simulink model "MCVT_Integration"  (R2020a)
settings: Solver=ode8  StartTime=0.0  StopTime=T_end  FixedStep=dt  RelTol=1e-3  EnableMultiTasking=on
values from neighbouring files (symbols this model uses):
  assumed_power_voltage = 1.25e2    (= 125)    [MCVT_Input_File.m]    (also in MCVT_Run_QuickCheck.m)
  battery_power_toggle = 1    [MCVT_Input_File.m]    (also in MCVT_Run_QuickCheck.m)
  battery_trickle_charge_param = 0.1    [MCVT_Input_File.m]    (also in MCVT_Run_QuickCheck.m)
  battery_trickle_leakage_param = 0.01    [MCVT_Input_File.m]    (also in MCVT_Run_QuickCheck.m)
  damage_case_id = 3    [MCVT_Input_File.m]    (also in MCVT_Run_QuickCheck.m = 1)
  dt = 1/fs    (= 0.001)    [MCVT_Run_QuickCheck.m]    (also in MCVT_Sim_Set.m)
  dt_2 = 1/fs_2    (= 0.001)    [MCVT_Run_QuickCheck.m]    (also in MCVT_Sim_Set.m)
  dt_3 = 1/fs_3    (= 0.001)    [MCVT_Run_QuickCheck.m]    (also in MCVT_Sim_Set.m)
  dt_5 = 1/fs_5    (= 0.005)    [MCVT_Run_QuickCheck.m]    (also in MCVT_Sim_Set.m)
  dt_8 = 1/fs_8    (= 0.05)    [MCVT_Run_QuickCheck.m]    (also in MCVT_Sim_Set.m)
  dt_9 = 1/fs_9    (= 0.001)    [MCVT_Run_QuickCheck.m]    (also in MCVT_Sim_Set.m)
  dust_met_duration = 3    [MCVT_Input_File.m]    (also in MCVT_Run_QuickCheck.m = 2)
  ECLSS_indoor_set_temperature = 298.15    [MCVT_Input_File.m]    (also in MCVT_Run_QuickCheck.m)
  energy_store_max_o = 150    [MCVT_Input_File.m]    (also in MCVT_Run_QuickCheck.m)
  energy_store_o = 120    [MCVT_Input_File.m]    (also in MCVT_Run_QuickCheck.m)
  fs = 1000    [MCVT_Run_QuickCheck.m]    (also in MCVT_Sim_Set.m)
  gain_charge_param = 1    [MCVT_Input_File.m]    (also in MCVT_Run_QuickCheck.m)
  ground_temperature = 373    [MCVT_Habitat_Design_Input_File.m]    (also in MCVT_Run_QuickCheck.m)
  I_max_charge = 800    [MCVT_Input_File.m]    (also in MCVT_Run_QuickCheck.m)
  I_max_discharge = 800    [MCVT_Input_File.m]    (also in MCVT_Run_QuickCheck.m)
  initial_pressure = 1e5    (= 100000)    [MCVT_Input_File.m]    (also in MCVT_Run_QuickCheck.m)
  initial_temperature = 295    [MCVT_Input_File.m]    (also in MCVT_Run_QuickCheck.m)
  interior_leak_rate = 0.00415    [MCVT_Input_File.m]    (also in MCVT_Run_QuickCheck.m)
  launch_dust_accum_start_time = 10    [MCVT_Input_File.m]    (also in MCVT_Run_QuickCheck.m = 2)
  launch_dust_accum_stop_time = 12    [MCVT_Input_File.m]    (also in MCVT_Run_QuickCheck.m = 3)
  meteorite_impact_time = 2    [MCVT_Input_File.m]    (also in MCVT_Run_QuickCheck.m = 1)
  nuclear_power_toggle = 1    [MCVT_Input_File.m]    (also in MCVT_Run_QuickCheck.m)
  print_interval = 30    [MCVT_Input_File.m]
  SOC_lower_thres = 0.5    [MCVT_Input_File.m]    (also in MCVT_Run_QuickCheck.m)
  SOC_upper_thres = 0.9    [MCVT_Input_File.m]    (also in MCVT_Run_QuickCheck.m)
  solar_power_toggle = 1    [MCVT_Input_File.m]    (also in MCVT_Run_QuickCheck.m)
  space_temperature = 4    [MCVT_Habitat_Design_Input_File.m]    (also in MCVT_Run_QuickCheck.m)
  Start_angle = 90    [MCVT_Input_File.m]    (also in MCVT_Run_QuickCheck.m)
undefined symbols (not defined in the file or in its folder): A_flow_restrictor, ACTIVITY_LOOKUP_TABLE, air, Aref, Bmap, Bmap2, Bmap3, comms, DiscIntNeverNeededParam, DmgSPL, dust_parameters, ECLSS_dust_damage_repair_time, ECLSS_heater_capacity, ECLSS_IPS_restrictor, ECLSS_IPS_setpointPressure, ECLSS_paint_damage_repair_time, ECLSS_radiatorLoop_discreteNumber, ECLSS_Vst, emptyActivity, expected_heat_add, FI, habitat, HIEM2SMM, HIEM2STM, HIEMDamageElements, impact_numbering, initial_SPLT_damage_index, input_air, input_Cond, input_CP, input_discretization, input_EV, input_Evap, input_radiatorLoop, K_ECLSS_dust_single, K_ECLSS_paint_single, K_Structure_damage_single, Kref, kW_to_W, meteoroidDustDep, mFRF, ndelays, nominalDustDep, noverlap, nuclear_toggle_gain, output_limits, panel_paint0, Ps0, reference_temperature, RepairTime_ECLSS_factor, Sensor_Location_SMM, SMM_T0, SMM_T1, SMM_T2, SMM_T3, SMM_T4, SMM_T4_0, SMM_T4_1, SMM_T4_2, solar_FDD_K ... (+73)
libraries: neural x350, simulink x39, xpcutilitieslib x7, xpcrtudplib x7, dspstat3 x1
(part 37 of 43)

== system MCVT_Integration/Sys3 - Power System/Power Generation Subsystem1/Nuclear Power Generation/SP-100 Power Generation/	Power Generation/Nominal Power/Sensor Models/Cold Junction Temperature Sensing Model/ Resistance Temperature Detector/Switch ON//OFF Control Block ==
  In1 : Inport
  In2 : Inport  Port=2
  Multiport Switch1 : MultiPortSwitch  Inputs=2
  Switch OFF : Subsystem
  Switch ON : Subsystem
  Out1 : Outport  VectorParamsAs1DForOutWhenUnconnected=off
  wires:
  Multiport Switch1/1 -> Out1/1
  In2/1 -> Switch ON/1(In1), Switch OFF/1(In1)
  In1/1 -> Multiport Switch1/1
  Switch ON/1(ON) -> Multiport Switch1/2
  Switch OFF/1(OFF) -> Multiport Switch1/3

== system MCVT_Integration/Sys3 - Power System/Power Generation Subsystem1/Nuclear Power Generation/SP-100 Power Generation/	Power Generation/Nominal Power/Sensor Models/Cold Junction Temperature Sensing Model1/ Resistance Temperature Detector/RTD with Self-heat Error ==
  Resistance Change with Error : Inport
  " Current in Circuit" : Constant  Value=0.001
  MATLAB Function : MATLAB Function  TreatAsAtomicUnit=on
  Voltage Output with Error : Outport  VectorParamsAs1DForOutWhenUnconnected=off
  wires:
  Resistance Change with Error/1 -> MATLAB Function/2(R)
  MATLAB Function/1(U) -> Voltage Output with Error/1
  " Current in Circuit"/1 -> MATLAB Function/1(I)

== system MCVT_Integration/Sys3 - Power System/Power Generation Subsystem1/Nuclear Power Generation/SP-100 Power Generation/	Power Generation/Nominal Power/Sensor Models/Cold Junction Temperature Sensing Model1/ Resistance Temperature Detector/RTD without Self-heat Error ==
  Resistance Change without Error : Inport
  " Current in Circuit" : Constant  Value=0.001
  MATLAB Function : MATLAB Function  TreatAsAtomicUnit=on
  Current in Circuit : Outport  VectorParamsAs1DForOutWhenUnconnected=off
  Voltage Output without Error : Outport  Port=2  VectorParamsAs1DForOutWhenUnconnected=off
  wires:
  Resistance Change without Error/1 -> MATLAB Function/2(R)
  MATLAB Function/2(U) -> Voltage Output without Error/1
  MATLAB Function/1(I) -> Current in Circuit/1
  " Current in Circuit"/1 -> MATLAB Function/1("I ")

== system MCVT_Integration/Sys3 - Power System/Power Generation Subsystem1/Nuclear Power Generation/SP-100 Power Generation/	Power Generation/Nominal Power/Sensor Models/Cold Junction Temperature Sensing Model1/ Resistance Temperature Detector/Self-heat Calculation ==
  note: Power = I 2 *R
  Real Temperature : Inport
  Resistance Change without Error : Inport  Port=2
  Current in Circuit : Inport  Port=3
  "  T vs R " : MATLAB Function  TreatAsAtomicUnit=on
  Add1 : Sum
  Divide1 : Product  Inputs=*/
  Gain : Gain  Gain=1000
  Product : Product
  Self Heating Constant (mW/℃) : Constant  Value=15
  Square : Math  Operator=square  SignedPower=on  SaturateOnIntegerOverflow=on
  Resistance Change with Error : Outport  VectorParamsAs1DForOutWhenUnconnected=off
  Self-heat Error : Outport  Port=2  VectorParamsAs1DForOutWhenUnconnected=off
  wires:
  Self Heating Constant (mW/℃)/1 -> Divide1/2
  Product/1 -> Divide1/1
  Add1/1 -> "  T vs R "/1(T)
  Divide1/1 -> Self-heat Error/1, Add1/2  "self_heat_error"
  Resistance Change without Error/1 -> Product/1
  "  T vs R "/1(R) -> Resistance Change with Error/1
  Real Temperature/1 -> Add1/1
  Gain/1 -> Square/1
  Square/1 -> Product/2
  Current in Circuit/1 -> Gain/1

== system MCVT_Integration/Sys3 - Power System/Power Generation Subsystem1/Nuclear Power Generation/SP-100 Power Generation/	Power Generation/Nominal Power/Sensor Models/Cold Junction Temperature Sensing Model1/ Resistance Temperature Detector/Switch ON//OFF Control Block ==
  In1 : Inport
  In2 : Inport  Port=2
  Multiport Switch1 : MultiPortSwitch  Inputs=2
  Switch OFF : Subsystem
  Switch ON : Subsystem
  Out1 : Outport  VectorParamsAs1DForOutWhenUnconnected=off
  wires:
  Multiport Switch1/1 -> Out1/1
  In2/1 -> Switch ON/1(In1), Switch OFF/1(In1)
  In1/1 -> Multiport Switch1/1
  Switch ON/1(ON) -> Multiport Switch1/2
  Switch OFF/1(OFF) -> Multiport Switch1/3

== system MCVT_Integration/Sys3 - Power System/Power Generation Subsystem1/Nuclear Power Generation/SP-100 Power Generation/	Power Generation/Nominal Power/Sensor Models/Cold Junction Temperature Sensing Model2/ Resistance Temperature Detector/RTD with Self-heat Error ==
  Resistance Change with Error : Inport
  " Current in Circuit" : Constant  Value=0.001
  MATLAB Function : MATLAB Function  TreatAsAtomicUnit=on
  Voltage Output with Error : Outport  VectorParamsAs1DForOutWhenUnconnected=off
  wires:
  " Current in Circuit"/1 -> MATLAB Function/1(I)
  MATLAB Function/1(U) -> Voltage Output with Error/1
  Resistance Change with Error/1 -> MATLAB Function/2(R)

== system MCVT_Integration/Sys3 - Power System/Power Generation Subsystem1/Nuclear Power Generation/SP-100 Power Generation/	Power Generation/Nominal Power/Sensor Models/Cold Junction Temperature Sensing Model2/ Resistance Temperature Detector/RTD without Self-heat Error ==
  Resistance Change without Error : Inport
  " Current in Circuit" : Constant  Value=0.001
  MATLAB Function : MATLAB Function  TreatAsAtomicUnit=on
  Current in Circuit : Outport  VectorParamsAs1DForOutWhenUnconnected=off
  Voltage Output without Error : Outport  Port=2  VectorParamsAs1DForOutWhenUnconnected=off
  wires:
  " Current in Circuit"/1 -> MATLAB Function/1("I ")
  MATLAB Function/1(I) -> Current in Circuit/1
  MATLAB Function/2(U) -> Voltage Output without Error/1
  Resistance Change without Error/1 -> MATLAB Function/2(R)

== system MCVT_Integration/Sys3 - Power System/Power Generation Subsystem1/Nuclear Power Generation/SP-100 Power Generation/	Power Generation/Nominal Power/Sensor Models/Cold Junction Temperature Sensing Model2/ Resistance Temperature Detector/Self-heat Calculation ==
  note: Power = I 2 *R
  Real Temperature : Inport
  Resistance Change without Error : Inport  Port=2
  Current in Circuit : Inport  Port=3
  "  T vs R " : MATLAB Function  TreatAsAtomicUnit=on
  Add1 : Sum
  Divide1 : Product  Inputs=*/
  Gain : Gain  Gain=1000
  Product : Product
  Self Heating Constant (mW/℃) : Constant  Value=15
  Square : Math  Operator=square  SignedPower=on  SaturateOnIntegerOverflow=on
  Resistance Change with Error : Outport  VectorParamsAs1DForOutWhenUnconnected=off
  Self-heat Error : Outport  Port=2  VectorParamsAs1DForOutWhenUnconnected=off
  wires:
  Current in Circuit/1 -> Gain/1
  Square/1 -> Product/2
  Gain/1 -> Square/1
  Real Temperature/1 -> Add1/1
  "  T vs R "/1(R) -> Resistance Change with Error/1
  Resistance Change without Error/1 -> Product/1
  Divide1/1 -> Add1/2, Self-heat Error/1
  Add1/1 -> "  T vs R "/1(T)
  Product/1 -> Divide1/1
  Self Heating Constant (mW/℃)/1 -> Divide1/2

== system MCVT_Integration/Sys3 - Power System/Power Generation Subsystem1/Nuclear Power Generation/SP-100 Power Generation/	Power Generation/Nominal Power/Sensor Models/Cold Junction Temperature Sensing Model2/ Resistance Temperature Detector/Switch ON//OFF Control Block ==
  In1 : Inport
  In2 : Inport  Port=2
  Multiport Switch1 : MultiPortSwitch  Inputs=2
  Switch OFF : Subsystem
  Switch ON : Subsystem
  Out1 : Outport  VectorParamsAs1DForOutWhenUnconnected=off
  wires:
  Switch OFF/1(OFF) -> Multiport Switch1/3
  Switch ON/1(ON) -> Multiport Switch1/2
  In1/1 -> Multiport Switch1/1
  In2/1 -> Switch OFF/1(In1), Switch ON/1(In1)
  Multiport Switch1/1 -> Out1/1

== system MCVT_Integration/Sys3 - Power System/Power Generation Subsystem1/Nuclear Power Generation/SP-100 Power Generation/	Power Generation/Nominal Power/Sensor Models/Cold Junction Temperature Sensing Model3/ Resistance Temperature Detector/RTD with Self-heat Error ==
  Resistance Change with Error : Inport
  " Current in Circuit" : Constant  Value=0.001
  MATLAB Function : MATLAB Function  TreatAsAtomicUnit=on
  Voltage Output with Error : Outport  VectorParamsAs1DForOutWhenUnconnected=off
  wires:
  Resistance Change with Error/1 -> MATLAB Function/2(R)
  MATLAB Function/1(U) -> Voltage Output with Error/1
  " Current in Circuit"/1 -> MATLAB Function/1(I)

== system MCVT_Integration/Sys3 - Power System/Power Generation Subsystem1/Nuclear Power Generation/SP-100 Power Generation/	Power Generation/Nominal Power/Sensor Models/Cold Junction Temperature Sensing Model3/ Resistance Temperature Detector/RTD without Self-heat Error ==
  Resistance Change without Error : Inport
  " Current in Circuit" : Constant  Value=0.001
  MATLAB Function : MATLAB Function  TreatAsAtomicUnit=on
  Current in Circuit : Outport  VectorParamsAs1DForOutWhenUnconnected=off
  Voltage Output without Error : Outport  Port=2  VectorParamsAs1DForOutWhenUnconnected=off
  wires:
  Resistance Change without Error/1 -> MATLAB Function/2(R)
  MATLAB Function/2(U) -> Voltage Output without Error/1
  MATLAB Function/1(I) -> Current in Circuit/1
  " Current in Circuit"/1 -> MATLAB Function/1("I ")

== system MCVT_Integration/Sys3 - Power System/Power Generation Subsystem1/Nuclear Power Generation/SP-100 Power Generation/	Power Generation/Nominal Power/Sensor Models/Cold Junction Temperature Sensing Model3/ Resistance Temperature Detector/Self-heat Calculation ==
  note: Power = I 2 *R
  Real Temperature : Inport
  Resistance Change without Error : Inport  Port=2
  Current in Circuit : Inport  Port=3
  "  T vs R " : MATLAB Function  TreatAsAtomicUnit=on
  Add1 : Sum
  Divide1 : Product  Inputs=*/
  Gain : Gain  Gain=1000
  Product : Product
  Self Heating Constant (mW/℃) : Constant  Value=15
  Square : Math  Operator=square  SignedPower=on  SaturateOnIntegerOverflow=on
  Resistance Change with Error : Outport  VectorParamsAs1DForOutWhenUnconnected=off
  Self-heat Error : Outport  Port=2  VectorParamsAs1DForOutWhenUnconnected=off
  wires:
  Self Heating Constant (mW/℃)/1 -> Divide1/2
  Product/1 -> Divide1/1
  Add1/1 -> "  T vs R "/1(T)
  Divide1/1 -> Self-heat Error/1, Add1/2
  Resistance Change without Error/1 -> Product/1
  "  T vs R "/1(R) -> Resistance Change with Error/1
  Real Temperature/1 -> Add1/1
  Gain/1 -> Square/1
  Square/1 -> Product/2
  Current in Circuit/1 -> Gain/1

== system MCVT_Integration/Sys3 - Power System/Power Generation Subsystem1/Nuclear Power Generation/SP-100 Power Generation/	Power Generation/Nominal Power/Sensor Models/Cold Junction Temperature Sensing Model3/ Resistance Temperature Detector/Switch ON//OFF Control Block ==
  In1 : Inport
  In2 : Inport  Port=2
  Multiport Switch1 : MultiPortSwitch  Inputs=2
  Switch OFF : Subsystem
  Switch ON : Subsystem
  Out1 : Outport  VectorParamsAs1DForOutWhenUnconnected=off
  wires:
  Multiport Switch1/1 -> Out1/1
  In2/1 -> Switch ON/1(In1), Switch OFF/1(In1)
  In1/1 -> Multiport Switch1/1
  Switch ON/1(ON) -> Multiport Switch1/2
  Switch OFF/1(OFF) -> Multiport Switch1/3

== system MCVT_Integration/Sys3 - Power System/Power Generation Subsystem1/Nuclear Power Generation/SP-100 Power Generation/	Power Generation/Nominal Power/Sensor Models/Cold Junction Temperature Sensing Model4/ Resistance Temperature Detector/RTD with Self-heat Error ==
  Resistance Change with Error : Inport
  " Current in Circuit" : Constant  Value=0.001
  MATLAB Function : MATLAB Function  TreatAsAtomicUnit=on
  Voltage Output with Error : Outport  VectorParamsAs1DForOutWhenUnconnected=off
  wires:
  " Current in Circuit"/1 -> MATLAB Function/1(I)
  MATLAB Function/1(U) -> Voltage Output with Error/1
  Resistance Change with Error/1 -> MATLAB Function/2(R)

== system MCVT_Integration/Sys3 - Power System/Power Generation Subsystem1/Nuclear Power Generation/SP-100 Power Generation/	Power Generation/Nominal Power/Sensor Models/Cold Junction Temperature Sensing Model4/ Resistance Temperature Detector/RTD without Self-heat Error ==
  Resistance Change without Error : Inport
  " Current in Circuit" : Constant  Value=0.001
  MATLAB Function : MATLAB Function  TreatAsAtomicUnit=on
  Current in Circuit : Outport  VectorParamsAs1DForOutWhenUnconnected=off
  Voltage Output without Error : Outport  Port=2  VectorParamsAs1DForOutWhenUnconnected=off
  wires:
  " Current in Circuit"/1 -> MATLAB Function/1("I ")
  MATLAB Function/1(I) -> Current in Circuit/1
  MATLAB Function/2(U) -> Voltage Output without Error/1
  Resistance Change without Error/1 -> MATLAB Function/2(R)

== system MCVT_Integration/Sys3 - Power System/Power Generation Subsystem1/Nuclear Power Generation/SP-100 Power Generation/	Power Generation/Nominal Power/Sensor Models/Cold Junction Temperature Sensing Model4/ Resistance Temperature Detector/Self-heat Calculation ==
  note: Power = I 2 *R
  Real Temperature : Inport
  Resistance Change without Error : Inport  Port=2
  Current in Circuit : Inport  Port=3
  "  T vs R " : MATLAB Function  TreatAsAtomicUnit=on
  Add1 : Sum
  Divide1 : Product  Inputs=*/
  Gain : Gain  Gain=1000
  Product : Product
  Self Heating Constant (mW/℃) : Constant  Value=15
  Square : Math  Operator=square  SignedPower=on  SaturateOnIntegerOverflow=on
  Resistance Change with Error : Outport  VectorParamsAs1DForOutWhenUnconnected=off
  Self-heat Error : Outport  Port=2  VectorParamsAs1DForOutWhenUnconnected=off
  wires:
  Current in Circuit/1 -> Gain/1
  Square/1 -> Product/2
  Gain/1 -> Square/1
  Real Temperature/1 -> Add1/1
  "  T vs R "/1(R) -> Resistance Change with Error/1
  Resistance Change without Error/1 -> Product/1
  Divide1/1 -> Add1/2, Self-heat Error/1
  Add1/1 -> "  T vs R "/1(T)
  Product/1 -> Divide1/1
  Self Heating Constant (mW/℃)/1 -> Divide1/2

== system MCVT_Integration/Sys3 - Power System/Power Generation Subsystem1/Nuclear Power Generation/SP-100 Power Generation/	Power Generation/Nominal Power/Sensor Models/Cold Junction Temperature Sensing Model4/ Resistance Temperature Detector/Switch ON//OFF Control Block ==
  In1 : Inport
  In2 : Inport  Port=2
  Multiport Switch1 : MultiPortSwitch  Inputs=2
  Switch OFF : Subsystem
  Switch ON : Subsystem
  Out1 : Outport  VectorParamsAs1DForOutWhenUnconnected=off
  wires:
  Switch OFF/1(OFF) -> Multiport Switch1/3
  Switch ON/1(ON) -> Multiport Switch1/2
  In1/1 -> Multiport Switch1/1
  In2/1 -> Switch OFF/1(In1), Switch ON/1(In1)
  Multiport Switch1/1 -> Out1/1

== system MCVT_Integration/Sys3 - Power System/Power Generation Subsystem1/Nuclear Power Generation/SP-100 Power Generation/	Power Generation/Sensor Models/Cold Junction Temperature Sensing Model/ Resistance Temperature Detector/Switch ON//OFF Control Block/Switch OFF ==
  In1 : Inport
  Clock1 : Clock
  MATLAB Function1 : MATLAB Function  TreatAsAtomicUnit=on
  No Signal : Scope
  OFF : Outport  VectorParamsAs1DForOutWhenUnconnected=off
  wires:
  In1/1 -> MATLAB Function1/1(u)
  Clock1/1 -> MATLAB Function1/2(Time)
  MATLAB Function1/1(y) -> OFF/1, No Signal/1

== system MCVT_Integration/Sys3 - Power System/Power Generation Subsystem1/Nuclear Power Generation/SP-100 Power Generation/	Power Generation/Sensor Models/Cold Junction Temperature Sensing Model/ Resistance Temperature Detector/Switch ON//OFF Control Block/Switch ON ==
  In1 : Inport
  Gain2 : Gain
  Signal Received : Scope
  ON : Outport  VectorParamsAs1DForOutWhenUnconnected=off
  wires:
  Gain2/1 -> ON/1, Signal Received/1
  In1/1 -> Gain2/1

== system MCVT_Integration/Sys3 - Power System/Power Generation Subsystem1/Nuclear Power Generation/SP-100 Power Generation/	Power Generation/Sensor Models/Cold Junction Temperature Sensing Model1/ Resistance Temperature Detector/Switch ON//OFF Control Block/Switch OFF ==
  In1 : Inport
  Clock1 : Clock
  MATLAB Function1 : MATLAB Function  TreatAsAtomicUnit=on
  No Signal : Scope
  OFF : Outport  VectorParamsAs1DForOutWhenUnconnected=off
  wires:
  In1/1 -> MATLAB Function1/1(u)
  Clock1/1 -> MATLAB Function1/2(Time)
  MATLAB Function1/1(y) -> OFF/1, No Signal/1

== system MCVT_Integration/Sys3 - Power System/Power Generation Subsystem1/Nuclear Power Generation/SP-100 Power Generation/	Power Generation/Sensor Models/Cold Junction Temperature Sensing Model1/ Resistance Temperature Detector/Switch ON//OFF Control Block/Switch ON ==
  In1 : Inport
  Gain2 : Gain
  Signal Received : Scope
  ON : Outport  VectorParamsAs1DForOutWhenUnconnected=off
  wires:
  Gain2/1 -> ON/1, Signal Received/1
  In1/1 -> Gain2/1

== system MCVT_Integration/Sys3 - Power System/Power Generation Subsystem1/Nuclear Power Generation/SP-100 Power Generation/	Power Generation/Sensor Models/Cold Junction Temperature Sensing Model2/ Resistance Temperature Detector/Switch ON//OFF Control Block/Switch OFF ==
  In1 : Inport
  Clock1 : Clock
  MATLAB Function1 : MATLAB Function  TreatAsAtomicUnit=on
  No Signal : Scope
  OFF : Outport  VectorParamsAs1DForOutWhenUnconnected=off
  wires:
  MATLAB Function1/1(y) -> No Signal/1, OFF/1
  Clock1/1 -> MATLAB Function1/2(Time)
  In1/1 -> MATLAB Function1/1(u)

== system MCVT_Integration/Sys3 - Power System/Power Generation Subsystem1/Nuclear Power Generation/SP-100 Power Generation/	Power Generation/Sensor Models/Cold Junction Temperature Sensing Model2/ Resistance Temperature Detector/Switch ON//OFF Control Block/Switch ON ==
  In1 : Inport
  Gain2 : Gain
  Signal Received : Scope
  ON : Outport  VectorParamsAs1DForOutWhenUnconnected=off
  wires:
  In1/1 -> Gain2/1
  Gain2/1 -> Signal Received/1, ON/1

== system MCVT_Integration/Sys3 - Power System/Power Generation Subsystem1/Nuclear Power Generation/SP-100 Power Generation/	Power Generation/Sensor Models/Cold Junction Temperature Sensing Model3/ Resistance Temperature Detector/Switch ON//OFF Control Block/Switch OFF ==
  In1 : Inport
  Clock1 : Clock
  MATLAB Function1 : MATLAB Function  TreatAsAtomicUnit=on
  No Signal : Scope
  OFF : Outport  VectorParamsAs1DForOutWhenUnconnected=off
  wires:
  In1/1 -> MATLAB Function1/1(u)
  Clock1/1 -> MATLAB Function1/2(Time)
  MATLAB Function1/1(y) -> OFF/1, No Signal/1

== system MCVT_Integration/Sys3 - Power System/Power Generation Subsystem1/Nuclear Power Generation/SP-100 Power Generation/	Power Generation/Sensor Models/Cold Junction Temperature Sensing Model3/ Resistance Temperature Detector/Switch ON//OFF Control Block/Switch ON ==
  In1 : Inport
  Gain2 : Gain
  Signal Received : Scope
  ON : Outport  VectorParamsAs1DForOutWhenUnconnected=off
  wires:
  Gain2/1 -> ON/1, Signal Received/1
  In1/1 -> Gain2/1

== system MCVT_Integration/Sys3 - Power System/Power Generation Subsystem1/Nuclear Power Generation/SP-100 Power Generation/	Power Generation/Sensor Models/Cold Junction Temperature Sensing Model4/ Resistance Temperature Detector/Switch ON//OFF Control Block/Switch OFF ==
  In1 : Inport
  Clock1 : Clock
  MATLAB Function1 : MATLAB Function  TreatAsAtomicUnit=on
  No Signal : Scope
  OFF : Outport  VectorParamsAs1DForOutWhenUnconnected=off
  wires:
  MATLAB Function1/1(y) -> No Signal/1, OFF/1
  Clock1/1 -> MATLAB Function1/2(Time)
  In1/1 -> MATLAB Function1/1(u)

== system MCVT_Integration/Sys3 - Power System/Power Generation Subsystem1/Nuclear Power Generation/SP-100 Power Generation/	Power Generation/Sensor Models/Cold Junction Temperature Sensing Model4/ Resistance Temperature Detector/Switch ON//OFF Control Block/Switch ON ==
  In1 : Inport
  Gain2 : Gain
  Signal Received : Scope
  ON : Outport  VectorParamsAs1DForOutWhenUnconnected=off
  wires:
  In1/1 -> Gain2/1
  Gain2/1 -> Signal Received/1, ON/1

== system MCVT_Integration/Sys3 - Power System/Power Generation Subsystem1/Nuclear Power Generation/SP-100 Power Generation/	Power Generation/Nominal Power/Sensor Models/Cold Junction Temperature Sensing Model/ Resistance Temperature Detector/Switch ON//OFF Control Block/Switch OFF ==
  In1 : Inport
  Clock1 : Clock
  MATLAB Function1 : MATLAB Function  TreatAsAtomicUnit=on
  No Signal : Scope
  OFF : Outport  VectorParamsAs1DForOutWhenUnconnected=off
  wires:
  In1/1 -> MATLAB Function1/1(u)
  Clock1/1 -> MATLAB Function1/2(Time)
  MATLAB Function1/1(y) -> OFF/1, No Signal/1

== system MCVT_Integration/Sys3 - Power System/Power Generation Subsystem1/Nuclear Power Generation/SP-100 Power Generation/	Power Generation/Nominal Power/Sensor Models/Cold Junction Temperature Sensing Model/ Resistance Temperature Detector/Switch ON//OFF Control Block/Switch ON ==
  In1 : Inport
  Gain2 : Gain
  Signal Received : Scope
  ON : Outport  VectorParamsAs1DForOutWhenUnconnected=off
  wires:
  Gain2/1 -> ON/1, Signal Received/1
  In1/1 -> Gain2/1

== system MCVT_Integration/Sys3 - Power System/Power Generation Subsystem1/Nuclear Power Generation/SP-100 Power Generation/	Power Generation/Nominal Power/Sensor Models/Cold Junction Temperature Sensing Model1/ Resistance Temperature Detector/Switch ON//OFF Control Block/Switch OFF ==
  In1 : Inport
  Clock1 : Clock
  MATLAB Function1 : MATLAB Function  TreatAsAtomicUnit=on
  No Signal : Scope
  OFF : Outport  VectorParamsAs1DForOutWhenUnconnected=off
  wires:
  In1/1 -> MATLAB Function1/1(u)
  Clock1/1 -> MATLAB Function1/2(Time)
  MATLAB Function1/1(y) -> OFF/1, No Signal/1

== system MCVT_Integration/Sys3 - Power System/Power Generation Subsystem1/Nuclear Power Generation/SP-100 Power Generation/	Power Generation/Nominal Power/Sensor Models/Cold Junction Temperature Sensing Model1/ Resistance Temperature Detector/Switch ON//OFF Control Block/Switch ON ==
  In1 : Inport
  Gain2 : Gain
  Signal Received : Scope
  ON : Outport  VectorParamsAs1DForOutWhenUnconnected=off
  wires:
  Gain2/1 -> ON/1, Signal Received/1
  In1/1 -> Gain2/1

== system MCVT_Integration/Sys3 - Power System/Power Generation Subsystem1/Nuclear Power Generation/SP-100 Power Generation/	Power Generation/Nominal Power/Sensor Models/Cold Junction Temperature Sensing Model2/ Resistance Temperature Detector/Switch ON//OFF Control Block/Switch OFF ==
  In1 : Inport
  Clock1 : Clock
  MATLAB Function1 : MATLAB Function  TreatAsAtomicUnit=on
  No Signal : Scope
  OFF : Outport  VectorParamsAs1DForOutWhenUnconnected=off
  wires:
  MATLAB Function1/1(y) -> No Signal/1, OFF/1
  Clock1/1 -> MATLAB Function1/2(Time)
  In1/1 -> MATLAB Function1/1(u)

== system MCVT_Integration/Sys3 - Power System/Power Generation Subsystem1/Nuclear Power Generation/SP-100 Power Generation/	Power Generation/Nominal Power/Sensor Models/Cold Junction Temperature Sensing Model2/ Resistance Temperature Detector/Switch ON//OFF Control Block/Switch ON ==
  In1 : Inport
  Gain2 : Gain
  Signal Received : Scope
  ON : Outport  VectorParamsAs1DForOutWhenUnconnected=off
  wires:
  In1/1 -> Gain2/1
  Gain2/1 -> Signal Received/1, ON/1

== system MCVT_Integration/Sys3 - Power System/Power Generation Subsystem1/Nuclear Power Generation/SP-100 Power Generation/	Power Generation/Nominal Power/Sensor Models/Cold Junction Temperature Sensing Model3/ Resistance Temperature Detector/Switch ON//OFF Control Block/Switch OFF ==
  In1 : Inport
  Clock1 : Clock
  MATLAB Function1 : MATLAB Function  TreatAsAtomicUnit=on
  No Signal : Scope
  OFF : Outport  VectorParamsAs1DForOutWhenUnconnected=off
  wires:
  In1/1 -> MATLAB Function1/1(u)
  Clock1/1 -> MATLAB Function1/2(Time)
  MATLAB Function1/1(y) -> OFF/1, No Signal/1

== system MCVT_Integration/Sys3 - Power System/Power Generation Subsystem1/Nuclear Power Generation/SP-100 Power Generation/	Power Generation/Nominal Power/Sensor Models/Cold Junction Temperature Sensing Model3/ Resistance Temperature Detector/Switch ON//OFF Control Block/Switch ON ==
  In1 : Inport
  Gain2 : Gain
  Signal Received : Scope
  ON : Outport  VectorParamsAs1DForOutWhenUnconnected=off
  wires:
  Gain2/1 -> ON/1, Signal Received/1
  In1/1 -> Gain2/1

== system MCVT_Integration/Sys3 - Power System/Power Generation Subsystem1/Nuclear Power Generation/SP-100 Power Generation/	Power Generation/Nominal Power/Sensor Models/Cold Junction Temperature Sensing Model4/ Resistance Temperature Detector/Switch ON//OFF Control Block/Switch OFF ==
  In1 : Inport
  Clock1 : Clock
  MATLAB Function1 : MATLAB Function  TreatAsAtomicUnit=on
  No Signal : Scope
  OFF : Outport  VectorParamsAs1DForOutWhenUnconnected=off
  wires:
  MATLAB Function1/1(y) -> No Signal/1, OFF/1
  Clock1/1 -> MATLAB Function1/2(Time)
  In1/1 -> MATLAB Function1/1(u)

== system MCVT_Integration/Sys3 - Power System/Power Generation Subsystem1/Nuclear Power Generation/SP-100 Power Generation/	Power Generation/Nominal Power/Sensor Models/Cold Junction Temperature Sensing Model4/ Resistance Temperature Detector/Switch ON//OFF Control Block/Switch ON ==
  In1 : Inport
  Gain2 : Gain
  Signal Received : Scope
  ON : Outport  VectorParamsAs1DForOutWhenUnconnected=off
  wires:
  In1/1 -> Gain2/1
  Gain2/1 -> Signal Received/1, ON/1
